simulink model "pasp_16i16c128s_part"  (Simulink 7.0)
settings: Solver=ode45  StartTime=0.0  StopTime=10  RelTol=1e-3
undefined symbols (not defined in the file or in its folder): pfb_coeff_gen_calc
libraries: xbsIndex_r4 x2019, casper_library x163, xps_library x21, gavrt_library x2
(part 1 of 21)
settings Model: Creator=tfiliba  LastModifiedBy=tfiliba
settings DebuggingCC: SaveWithParameterizedLinksMsg=none  SignalResolutionControl=TryResolveAllWithWarning  MultiTaskDSMMsg=warning  MultiTaskCondExecSysMsg=none  StrictBusMsg=None
settings RTWCC: IncludeERTFirstTime=on
settings GRTTargetCC: TargetFcnLib=ansi_tfl_tmw.mat

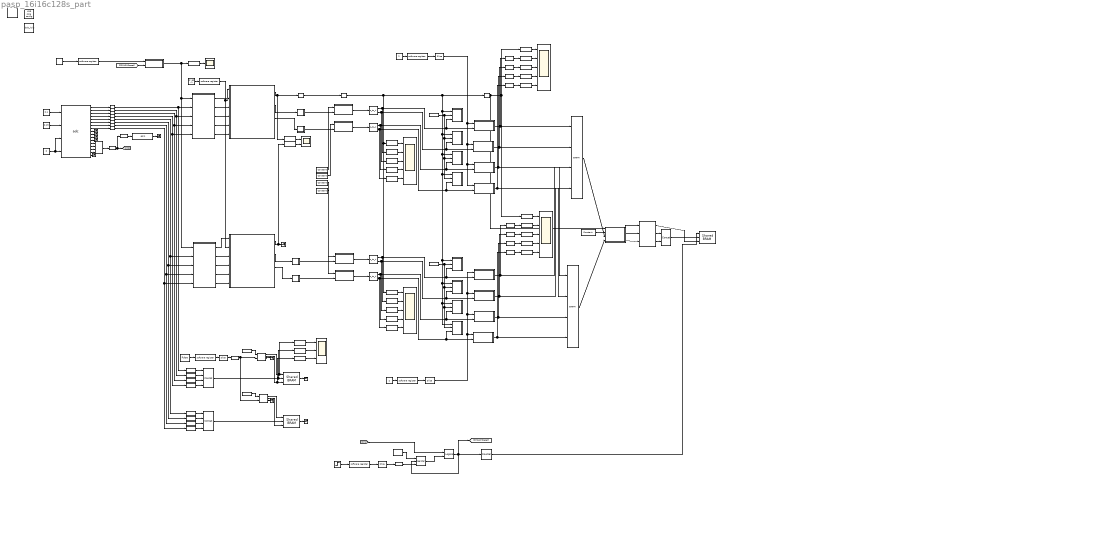
== system pasp_16i16c128s_part ==
  note: left
  note: Coherent-dedispersion Pulsar Machine
      | Nancay Radio Telescope
      | Peter McMahon (CASPER/meerKAT)
      | August 2008
      | Version 1.0
  note: ADC Scope
      | Outputs raw bits from ADC so you can measure voltage levels
  note: n * LCM(reoder_order=2)*(taps*clksperFFT=2*64=128)=256
  note: Pol 1
  note: Pol 2
  note: Real sampling at 800MSa/sec
      | to give B/W of 0-400MHz
  note: ADC Scope
      | Outputs raw bits from ADC so you can measure voltage levels
  note: ARM/PPS Reset Circuit
      | 1. User sets ARM high at half-second
      | 2. 1PPS arrives.
      | 3. PPSARMReset signal goes high for one clock on the 1PPS
      | 4. ARM is reset, and won't go high again until user brings it low then high
  note: Unsigned, 18-bits, b.p. at 12
      | Coefficient by which to scale the output of the FFT.
  note: Selects which 8 bits from 18 are outputted.
      | 0 is lowest 8-bits: bits 0-7 (inclusive)
      | 1: bits 4-9
      | 2: bits 8-15
      | 3 is highest 8-bits: bits 10-17
  note: Extract 8 bits from 18
  note: Extract 8 bits from 18
  note: Delay is here for timing purposes:
      | otherwise adc_sync fails timing.
  " System Generator" : xbsIndex_r4/ System Generator  Tag=genX  SystemSampleTime=-1  xilinxfamily=virtex2p  part=xc2vp50  speed=-7  package=ff1152  synthesis_tool=XST  clock_wrapper=Clock Enables  directory=./pasp_16i16c128s_part/sysgen  testbench=off  simulink_period=1  sysclk_period=5  dcm_input_clock_period=100  incr_netlist=off  trim_vbits=Everywhere in SubSystem  dbl_ovrd=According to Block Masks  core_generation=According to Block Masks  run_coregen=off  deprecated_control=off  eval_field=0  block_type=sysgen
      settings: block_version=10.1.3
  Concat1 : xbsIndex_r4/Concat  num_inputs=2  dbl_ovrd=off  block_type=concat
      settings: block_version=10.1.3
  Constant{,1,3..4} (4 alike) : xbsIndex_r4/Constant  arith_type=Unsigned  const=1  n_bits=18  bin_pt=12  explicit_period=on  period=1  equ=P=C  opselect=C  inp2=PCIN>>17  opr=+  inp1=P  carry=CIN  dbl_ovrd=off  block_type=constant
  Constant2 : Constant  Value=2  OutDataType=sfix(16)  OutScaling=2^0
  Constant5 : Constant  Value=2^32 - 1  OutDataType=sfix(16)  OutScaling=2^0
  Constant6 : Constant  Value=2  OutDataType=sfix(16)  OutScaling=2^0
  Gateway Out{1..5,8,10..19,21..30} (26 alike) : xbsIndex_r4/Gateway Out  hdl_port=off  timing_constraint=None  locs_specified=off  LOCs={}  block_type=gatewayout
      settings: infoedit="Gateway out block.  Converts Xilinx fixed point inputs into ouputs of type Simulink integer, double, or fixed point.<P><P>Hardware notes:  In hardware these blocks become top level output ports or are discarded, depending on how they are configured."  xl_area=[0, 0, 0, 0, 0, 0, 0]  block_version=10.1.3
  Reinterpret{,1..3,8..11} (8 alike) : xbsIndex_r4/Reinterpret  force_arith_type=on  arith_type="Signed  (2's comp)"  force_bin_pt=on  bin_pt=0  block_type=reinterpret
      settings: infoedit="Changes signal type without altering the binary representation.   You can changed the signal between signed and unsigned, and relocate the binary point.<P><P>Hardware notes: In hardware this block costs nothing.<P><P>Example:  Suppose the input is 6 bits wide, signed, with 2 fractional bits, and the output is forced to unsigned with 0 fractional bits.  Then an input of -2.0 (1110.00 in binary 2's complement) becomes an output of 56 (111000 in binary)."  block_version=10.1.3
  Scope : Scope  NumInputPorts=3
      settings: YMin=-5~-5~-5  YMax=5~5~5  SaveName=ScopeData8
  Scope1 : Scope  NumInputPorts=2
      settings: YMin=-1~-1  YMax=1~1  SaveName=ScopeData7
  Scope2 : Scope
      settings: YMin=-1  YMax=1  SaveName=ScopeData9
  Scope{3..6} (4 alike) : Scope  NumInputPorts=5
      Scope3 settings: YMin=-1~-2.5e-005~-1.75e-005~-2.5e-005~-8e-006  YMax=1~0~0~0~0  SaveName=ScopeData3
      Scope4 settings: YMin=-1~-1~-1~-1~-1  YMax=1~0~0~0~0  SaveName=ScopeData10
      Scope5 settings: YMin=-1~-2.5e-005~-1.75e-005~-2.5e-005~-8e-006  YMax=1~0~0~0~0  SaveName=ScopeData5
      Scope6 settings: YMin=-1~-1~-1~-1~-1  YMax=1~0~0~0~0  SaveName=ScopeData11
  Slice10 : xbsIndex_r4/Slice  nbits=2  boolean_output=off  mode=Lower Bit Location + Width  bit1=0  base1=MSB of Input  bit0=0  base0=LSB of Input  dbl_ovrd=off  block_type=slice
      settings: infoedit="Extracts a given range of bits from each input sample and presents it at the output.  The output type is ordinarily unsigned with binary point at zero, but can be Boolean when the slice is one bit wide.<P><P>Hardware notes: In hardware this block costs nothing."  block_version=10.1.3
  Slice2 : xbsIndex_r4/Slice  nbits=2  boolean_output=off  mode=Lower Bit Location + Width  bit1=0  base1=MSB of Input  bit0=0  base0=LSB of Input  dbl_ovrd=off  block_type=slice
      settings: infoedit="Extracts a given range of bits from each input sample and presents it at the output.  The output type is ordinarily unsigned with binary point at zero, but can be Boolean when the slice is one bit wide.<P><P>Hardware notes: In hardware this block costs nothing."  block_version=10.1.3
  Slice8 : xbsIndex_r4/Slice  nbits=1  boolean_output=on  mode=Lower Bit Location + Width  bit1=0  base1=MSB of Input  bit0=0  base0=LSB of Input  dbl_ovrd=off  block_type=slice
      settings: infoedit="Extracts a given range of bits from each input sample and presents it at the output.  The output type is ordinarily unsigned with binary point at zero, but can be Boolean when the slice is one bit wide.<P><P>Hardware notes: In hardware this block costs nothing."  block_version=10.1.3
  Terminator{,1..9,11} (11 alike) : Terminator
  XSG core config : xps_library/XSG core config  Tag=xps:xsg  SystemSampleTime=-1  hw_sys=iBOB  ibob_linux=off  clk_src=adc0_clk  iBOB_clk_src=adc0_clk  BEE2_ctrl_clk_src=sys_clk  BEE2_usr_clk_src=sys_clk  ROACH_clk_src=sys_clk  CORR_clk_src=sys_clk  gpio_clk_io_group=iBOB:sma  gpio_clk_bit_index=0  clk_rate=200  sample_period=1  synthesis_tool=XST  mpc_type=powerpc405
  adc : xps_library/adc  Tag=xps:adc  SystemSampleTime=-1  adc_brd=adc0  adc_clk_rate=800  adc_interleave=off  sample_period=1
  adc_data_delay{1..8} (8 alike) : xbsIndex_r4/Delay  en=off  latency=1  dbl_ovrd=off  reg_retiming=on  block_type=delay
      settings: xl_area=[0, 0, 0, 0, 0, 0, 0]
  bitselect_1 : Subsystem
  bitselect_2 : Subsystem
  bitselect_3 : Subsystem
  bitselect_4 : Subsystem
  bitselect_5 : Subsystem
  bitselect_6 : Subsystem
  bitselect_7 : Subsystem
  bitselect_8 : Subsystem
  bram_adcscope_1 : xps_library/Shared BRAM  Tag=xps:bram  SystemSampleTime=-1  arith_type="Signed  (2's comp)"  addr_width=11  data_width=32  data_bin_pt=0  init_vals=[1:2^11]  sample_rate=1
      link override convert_din: arith_type="Signed  (2's comp)"
      link override mem/sim_data_in: arith_type="Signed  (2's comp)"
      link override pasp_16i16c128s_part_bram_adcscope_1_data_out: arith_type="Signed  (2's comp)"
      link override pasp_16i16c128s_pasp_bram_adcscope_1_data_out: arith_type="Signed  (2's comp)"
  bram_adcscope_2 : xps_library/Shared BRAM  Tag=xps:bram  SystemSampleTime=-1  arith_type="Signed  (2's comp)"  addr_width=11  data_width=32  data_bin_pt=0  init_vals=[1:2^11]  sample_rate=1
      link override convert_din: arith_type="Signed  (2's comp)"
      link override mem/sim_data_in: arith_type="Signed  (2's comp)"
      link override pasp_16i16c128s_part_bram_adcscope_2_data_out: arith_type="Signed  (2's comp)"
      link override pasp_16i16c128s_pasp_bram_adcscope_1_data_out: arith_type="Signed  (2's comp)"
  bram_adcscope_3 : xps_library/Shared BRAM  Tag=xps:bram  SystemSampleTime=-1  arith_type="Signed  (2's comp)"  addr_width=11  data_width=32  data_bin_pt=0  init_vals=[1:2^11]  sample_rate=1
      link override convert_din: arith_type="Signed  (2's comp)"
      link override mem/sim_data_in: arith_type="Signed  (2's comp)"
      link override pasp_16i16c128s_part_bram_adcscope_3_data_out: arith_type="Signed  (2's comp)"
      link override pasp_16i16c128s_pasp_bram_adcscope_2_data_out: arith_type="Signed  (2's comp)"
  bs_delay : xbsIndex_r4/Delay  en=off  latency=1  dbl_ovrd=off  reg_retiming=off  block_type=delay
      settings: xl_area=[0, 0, 0, 0, 0, 0, 0]
  c_to_ri{1..4} (4 alike) : casper_library/Misc/c_to_ri  SystemSampleTime=-1  n_bits=18  bin_pt=17
      settings: AttributesFormatString=18_17 r/i
  cns_adcscope_1 : xbsIndex_r4/Constant  arith_type=Boolean  const=1  n_bits=1  bin_pt=0  explicit_period=on  period=1  equ=P=C  opselect=C  inp2=PCIN>>17  opr=+  inp1=P  carry=CIN  dbl_ovrd=off  block_type=constant
  cns_adcscope_2 : xbsIndex_r4/Constant  arith_type=Boolean  const=1  n_bits=1  bin_pt=0  explicit_period=on  period=1  equ=P=C  opselect=C  inp2=PCIN>>17  opr=+  inp1=P  carry=CIN  dbl_ovrd=off  block_type=constant
  cns_reorder : xbsIndex_r4/Constant  arith_type=Boolean  const=1  n_bits=16  bin_pt=14  explicit_period=on  period=1  equ=P=C  opselect=C  inp2=PCIN>>17  opr=+  inp1=P  carry=CIN  dbl_ovrd=off  block_type=constant
  cns_reset : xbsIndex_r4/Constant  arith_type=Boolean  const=1  n_bits=1  bin_pt=0  explicit_period=on  period=1  equ=P=C  opselect=C  inp2=PCIN>>17  opr=+  inp1=P  carry=CIN  dbl_ovrd=off  block_type=constant
  cns_scopes_en0 : xbsIndex_r4/Constant  arith_type=Boolean  const=1  n_bits=1  bin_pt=0  explicit_period=on  period=1  equ=P=C  opselect=C  inp2=PCIN>>17  opr=+  inp1=P  carry=CIN  dbl_ovrd=off  block_type=constant
  cns_scopes_en1 : xbsIndex_r4/Constant  arith_type=Boolean  const=1  n_bits=1  bin_pt=0  explicit_period=on  period=1  equ=P=C  opselect=C  inp2=PCIN>>17  opr=+  inp1=P  carry=CIN  dbl_ovrd=off  block_type=constant
  cns_sync_period : Constant  Value=10240  OutDataType=sfix(16)  OutScaling=2^0
  cnt_adcscope_1 : casper_library/Misc/freeze_cntr  SystemSampleTime=-1  CounterBits=11
  cnt_adcscope_2 : casper_library/Misc/freeze_cntr  SystemSampleTime=-1  CounterBits=11
  coeff_delay : xbsIndex_r4/Delay  en=off  latency=6  dbl_ovrd=off  reg_retiming=on  block_type=delay
      settings: xl_area=[0, 0, 0, 0, 0, 0, 0]
  concat_adcscope_1 : xbsIndex_r4/Concat  num_inputs=4  dbl_ovrd=off  block_type=concat
      settings: block_version=10.1.3
  concat_adcscope_3 : xbsIndex_r4/Concat  num_inputs=4  dbl_ovrd=off  block_type=concat
      settings: block_version=10.1.3
  cram0 : gavrt_library/cram  SystemSampleTime=-1  num_slice=4
  cram1 : gavrt_library/cram  SystemSampleTime=-1  num_slice=4
  fft_delay : xbsIndex_r4/Delay  en=off  latency=4  dbl_ovrd=off  reg_retiming=off  block_type=delay
      settings: xl_area=[0, 0, 0, 0, 0, 0, 0]
  fft_wideband_real : Subsystem  AncestorBlock=casper_library/FFTs/fft_wideband_real  mask(fft_wideband_real){FFTSize=5, input_bit_width=18, coeff_bit_width=18, n_inputs=2, quantization=Truncate, overflow=Wrap, add_latency=2, mult_latency=3, bram_latency=2, arch=Virtex2Pro, opt_target=Multipliers, coeffs_bit_limit=2, delays_bit_limit=8, specify_mult=on, mult_spec=[1 1 1 1 1]}
      mask prompts: FFTSize "Size of FFT: (2^?)"; input_bit_width "Input/Output Bit Width"; coeff_bit_width "Coefficient Bit Width"; n_inputs "Number of Simultaneous Inputs: (2^?)"; quantization "Quantization Behavior"; overflow "Overflow Behavior"; add_latency "Add Latency"; mult_latency "Mult Latency"; bram_latency "BRAM Latency"; arch "Architecture"; opt_target "Optimisation Target"; coeffs_bit_limit "Number bits above which to store stage's coefficients in BRAM (2^? bits)"; delays_bit_limit "Number bits above which to store stage's delays in BRAM (2^? bits)"; specify_mult "Specify multiplier use (behavioral HDL if not specified)"; mult_spec "Multiplier specification (0=core, 1=embedded, 2=behavioural) (left=1st stage)"
      mask init:
      | fft_wideband_real_init(gcb,...
      |     'FFTSize', FFTSize,...
      |     'input_bit_width', input_bit_width,...
      |     'coeff_bit_width', coeff_bit_width,...
      |     'n_inputs', n_inputs,...
      |     'quantization', quantization,...
      |     'overflow', overflow,...
      |     'add_latency', add_latency,...
      |     'mult_latency', mult_latency,...
      |     'bram_latency', bram_latency,...
      |     'arch', arch, ...
      |     'opt_target', opt_target, ...
      |     'coeffs_bit_limit', coeffs_bit_limit, ...
      |     'delays_bit_limit', delays_bit_limit, ...    
      |     'specify_mult', specify_mult,...
      |     'mult_spec', mult_spec);
      settings: AttributesFormatString=FFTSize=5
      mask Help: eval('xlWeb([getenv(''MLIB_ROOT''), ''\doc\htmlblocks\sp_fft_wideband_real\sp_fft_wideband_real.html''])')
      mask StyleString: edit,edit,edit,edit,popup(Truncate|Round  (unbiased: +/- Inf)|Round  (unbiased: Even Values)),popup(Wrap|Saturate|Error),edit,edit,edit,popup(Virtex2Pro|Virtex5),popup(Logic|Multipliers),edit,edit,checkbox,edit
      mask CallbackString:
      | % fft_size = eval(get_param(gcb, 'FFTSize'));
      | % old_vec = eval(get_param(gcb, 'mult_spec'));
      | 
      | % vec = 2.*ones(1,fft_size);
      | % old_size = length(old_vec);
      | 
      | % if(old_size <= fft_size),
      | %    size = old_size;
      | % else,
      | %     size = fft_size;
      | % end
      | 
      | % for n = 1:size, vec(n) = old_vec(n); end
      | % set_param(gcb, 'mult_spec', mat2str(vec));|||||||||||||specify_mult = get_param(gcb,'specify_mult');
      | visibs = get_param(gcb, 'MaskVisibilities');
      | enables = get_param(gcb, 'MaskEnables');
      | enables{15} = 'on';
      | if( strcmp(specify_mult,'off')), visibs{15} = 'off';
      | else, visibs{15} = 'on';
      | end
      | set_param(gcb, 'MaskEnables', enables);
      | set_param(gcb, 'MaskVisibilities', visibs);
      | |% vec = eval( get_param(gcb, 'mult_spec'));
      | % vec_size = length(vec);
      | % fft_size = eval( get_param(gcb, 'FFTSize'));
      | % new_vec = 2.*ones(1, fft_size);
      | 
      | % if(vec_size <= fft_size), size = vec_size;
      | % else size = fft_size;
      | % end
      | 
      | % for n=1:size
      | %     new_vec(n) = vec(n);
      | % end
      | % set_param(gcb, 'mult_spec', mat2str(new_vec));
  fft_wideband_real1 : Subsystem  AncestorBlock=casper_library/FFTs/fft_wideband_real  mask(fft_wideband_real){FFTSize=5, input_bit_width=18, coeff_bit_width=18, n_inputs=2, quantization=Truncate, overflow=Wrap, add_latency=2, mult_latency=3, bram_latency=2, arch=Virtex2Pro, opt_target=Multipliers, coeffs_bit_limit=2, delays_bit_limit=8, specify_mult=on, mult_spec=[1 1 1 1 1]}
      mask prompts: FFTSize "Size of FFT: (2^?)"; input_bit_width "Input/Output Bit Width"; coeff_bit_width "Coefficient Bit Width"; n_inputs "Number of Simultaneous Inputs: (2^?)"; quantization "Quantization Behavior"; overflow "Overflow Behavior"; add_latency "Add Latency"; mult_latency "Mult Latency"; bram_latency "BRAM Latency"; arch "Architecture"; opt_target "Optimisation Target"; coeffs_bit_limit "Number bits above which to store stage's coefficients in BRAM (2^? bits)"; delays_bit_limit "Number bits above which to store stage's delays in BRAM (2^? bits)"; specify_mult "Specify multiplier use (behavioral HDL if not specified)"; mult_spec "Multiplier specification (0=core, 1=embedded, 2=behavioural) (left=1st stage)"
      mask init: same as pasp_16i16c128s_part/fft_wideband_real
      settings: AttributesFormatString=FFTSize=5
      mask Help: eval('xlWeb([getenv(''MLIB_ROOT''), ''\doc\htmlblocks\sp_fft_wideband_real\sp_fft_wideband_real.html''])')
      mask StyleString: edit,edit,edit,edit,popup(Truncate|Round  (unbiased: +/- Inf)|Round  (unbiased: Even Values)),popup(Wrap|Saturate|Error),edit,edit,edit,popup(Virtex2Pro|Virtex5),popup(Logic|Multipliers),edit,edit,checkbox,edit
      mask CallbackString:
      | % fft_size = eval(get_param(gcb, 'FFTSize'));
      | % old_vec = eval(get_param(gcb, 'mult_spec'));
      | 
      | % vec = 2.*ones(1,fft_size);
      | % old_size = length(old_vec);
      | 
      | % if(old_size <= fft_size),
      | %    size = old_size;
      | % else,
      | %     size = fft_size;
      | % end
      | 
      | % for n = 1:size, vec(n) = old_vec(n); end
      | % set_param(gcb, 'mult_spec', mat2str(vec));|||||||||||||specify_mult = get_param(gcb,'specify_mult');
      | visibs = get_param(gcb, 'MaskVisibilities');
      | enables = get_param(gcb, 'MaskEnables');
      | enables{15} = 'on';
      | if( strcmp(specify_mult,'off')), visibs{15} = 'off';
      | else, visibs{15} = 'on';
      | end
      | set_param(gcb, 'MaskEnables', enables);
      | set_param(gcb, 'MaskVisibilities', visibs);
      | |% vec = eval( get_param(gcb, 'mult_spec'));
      | % vec_size = length(vec);
      | % fft_size = eval( get_param(gcb, 'FFTSize'));
      | % new_vec = 2.*ones(1, fft_size);
      | 
      | % if(vec_size <= fft_size), size = vec_size;
      | % else size = fft_size;
      | % end
      | 
      | % for n=1:size
      | %     new_vec(n) = vec(n);
      | % end
      | % set_param(gcb, 'mult_spec', mat2str(new_vec));
  force_adc_i{0..3,8..11} (8 alike) : xbsIndex_r4/Reinterpret  force_arith_type=on  arith_type=Unsigned  force_bin_pt=on  bin_pt=0  block_type=reinterpret
      settings: infoedit="Changes signal type without altering the binary representation.   You can changed the signal between signed and unsigned, and relocate the binary point.<P><P>Hardware notes: In hardware this block costs nothing.<P><P>Example:  Suppose the input is 6 bits wide, signed, with 2 fractional bits, and the output is forced to unsigned with 0 fractional bits.  Then an input of -2.0 (1110.00 in binary 2's complement) becomes an output of 56 (111000 in binary)."  block_version=10.1.3
  ibob_lwip : xps_library/ibob_lwip  SystemSampleTime=-1
      link override macbits/convert: arith_type=Unsigned  n_bits=32  bin_pt=0
      link override machdr/pasp_16i16c128s_ibob_lwip_machdr_gateway: arith_type=Unsigned  n_bits=20  bin_pt=0  period=sample_period
      link override machdr/pasp_16i16c128s_part_ibob_lwip_machdr_gateway: arith_type=Unsigned  n_bits=20  bin_pt=0  period=sample_period
  led2_pps : xps_library/gpio  Tag=xps:gpio  SystemSampleTime=-1  io_group=iBOB:led  io_dir=out  arith_type=Boolean  bitwidth=1  bin_pt=0  bit_index=3  sample_period=1  use_single_ended=off  use_ddr=off  reg_iob=on  reg_clk_phase=0  termination=None
      link override convert: arith_type=Boolean  n_bits=1  bin_pt=0
  log_arm_pps : xbsIndex_r4/Logical  logical_function=AND  inputs=2  en=off  latency=0  precision=Full  arith_type=Unsigned  n_bits=8  bin_pt=2  align_bp=on  dbl_ovrd=off  block_type=logical
      settings: xl_area=[0, 0, 0, 0, 0, 0, 0]
  pfb_fir_real : Subsystem  AncestorBlock=casper_library/PFBs/pfb_fir_real  mask(pfb_fir_real){PFBSize=5, TotalTaps=2, WindowType=hamming, n_inputs=2, MakeBiplex=0, BitWidthIn=8, BitWidthOut=18, CoeffBitWidth=8, CoeffDistMem=0, add_latency=1, mult_latency=2, bram_latency=2, quantization=Truncate, fwidth=1, specify_mult=off, mult_spec=[0 1]}
      mask prompts: PFBSize "Size of PFB: (2^? pnts)"; TotalTaps "Total Number of Taps:"; WindowType "Windowing Function:"; n_inputs "Number of Simultaneous Inputs: (2^?)"; MakeBiplex "Make Biplex"; BitWidthIn "Input Bitwidth:"; BitWidthOut "Output Bitwidth:"; CoeffBitWidth "Coefficient Bitwidth:"; CoeffDistMem "Use Distributed Memory for Coeffs"; add_latency "Add Latency"; mult_latency "Mult Latency"; bram_latency "BRAM Latency"; quantization "Quantization Behavior"; fwidth "Bin Width Scaling (normal=1)"; specify_mult "Specify multiplier use (embedded if not specified)"; mult_spec "Multiplier specification (0=core, 1=embedded, 2=behavioural) (left=1st tap)"
      mask init:
      | pfb_fir_real_init(gcb,...
      |     'PFBSize', PFBSize,...
      |     'TotalTaps', TotalTaps,...
      |     'WindowType', WindowType,...
      |     'n_inputs', n_inputs,...
      |     'MakeBiplex', MakeBiplex,...
      |     'BitWidthIn', BitWidthIn,...
      |     'BitWidthOut', BitWidthOut,...
      |     'CoeffBitWidth', CoeffBitWidth,...
      |     'CoeffDistMem', CoeffDistMem,...
      |     'add_latency', add_latency,...
      |     'mult_latency', mult_latency,...
      |     'bram_latency', bram_latency,...
      |     'quantization', quantization,...
      |     'fwidth', fwidth,...
      |     'specify_mult', specify_mult,...
      |     'mult_spec', mult_spec);
      settings: AttributesFormatString=taps=2, add_latency=1
      mask Help: eval('xlWeb([getenv(''MLIB_ROOT''), ''\doc\htmlblocks\sp_pfb_fir_real\sp_pfb_fir_real.html''])')
      mask StyleString: edit,edit,popup(bartlett|barthannwin|blackman|blackmanharris|bohamwin|chebwin|flattopwin|gausswin|hamming|hann|kaiser|nuttallwin|parzenwin|rectwin|tukeywin|triang|userwindow),edit,edit,edit,edit,edit,edit,edit,edit,edit,popup(Truncate|Round  (unbiased: +/- Inf)|Round  (unbiased: Even Values)),edit,checkbox,edit
      mask CallbackString:
      | |num_taps = eval(get_param(gcb, 'TotalTaps'));
      | old_vec = eval(get_param(gcb, 'mult_spec'));
      | 
      | vec = ones(1,num_taps);
      | old_size = length(old_vec);
      | 
      | if(old_size <= num_taps),
      |     size = old_size;
      | else,
      |     size = num_taps;
      | end
      | 
      | for n = 1:size, vec(n) = old_vec(n); end
      | set_param(gcb, 'mult_spec', mat2str(vec));|||||||||||||specify_mult = get_param(gcb,'specify_mult');
      | if( strcmp(specify_mult,'off')),
      |     set_param(gcb, 'MaskEnables', {'on','on','on','on','on','on','on','on','on','on','on','on','on','on','on','off'});
      | else
      |     set_param(gcb, 'MaskEnables', {'on','on','on','on','on','on','on','on','on','on','on','on','on','on','on','on'});
      | end
      | 
      | |vec = eval( get_param(gcb, 'mult_spec'));
      | vec_size = length(vec);
      | num_taps = eval( get_param(gcb, 'TotalTaps'));
      | new_vec = ones(1, num_taps);
      | 
      | if(vec_size <= num_taps), size = vec_size;
      | else size = num_taps;
      | end
      | 
      | for n=1:size
      |     new_vec(n) = vec(n);
      | end
      | set_param(gcb, 'mult_spec', mat2str(new_vec));
  pfb_fir_real1 : Subsystem  AncestorBlock=casper_library/PFBs/pfb_fir_real  mask(pfb_fir_real){PFBSize=5, TotalTaps=2, WindowType=hamming, n_inputs=2, MakeBiplex=0, BitWidthIn=8, BitWidthOut=18, CoeffBitWidth=8, CoeffDistMem=0, add_latency=1, mult_latency=2, bram_latency=2, quantization=Truncate, fwidth=1, specify_mult=off, mult_spec=[0 1]}
      mask prompts: PFBSize "Size of PFB: (2^? pnts)"; TotalTaps "Total Number of Taps:"; WindowType "Windowing Function:"; n_inputs "Number of Simultaneous Inputs: (2^?)"; MakeBiplex "Make Biplex"; BitWidthIn "Input Bitwidth:"; BitWidthOut "Output Bitwidth:"; CoeffBitWidth "Coefficient Bitwidth:"; CoeffDistMem "Use Distributed Memory for Coeffs"; add_latency "Add Latency"; mult_latency "Mult Latency"; bram_latency "BRAM Latency"; quantization "Quantization Behavior"; fwidth "Bin Width Scaling (normal=1)"; specify_mult "Specify multiplier use (embedded if not specified)"; mult_spec "Multiplier specification (0=core, 1=embedded, 2=behavioural) (left=1st tap)"
      mask init: same as pasp_16i16c128s_part/pfb_fir_real
      settings: AttributesFormatString=taps=2, add_latency=1
      mask Help: eval('xlWeb([getenv(''MLIB_ROOT''), ''\doc\htmlblocks\sp_pfb_fir_real\sp_pfb_fir_real.html''])')
      mask StyleString: edit,edit,popup(bartlett|barthannwin|blackman|blackmanharris|bohamwin|chebwin|flattopwin|gausswin|hamming|hann|kaiser|nuttallwin|parzenwin|rectwin|tukeywin|triang|userwindow),edit,edit,edit,edit,edit,edit,edit,edit,edit,popup(Truncate|Round  (unbiased: +/- Inf)|Round  (unbiased: Even Values)),edit,checkbox,edit
      mask CallbackString:
      | |num_taps = eval(get_param(gcb, 'TotalTaps'));
      | old_vec = eval(get_param(gcb, 'mult_spec'));
      | 
      | vec = ones(1,num_taps);
      | old_size = length(old_vec);
      | 
      | if(old_size <= num_taps),
      |     size = old_size;
      | else,
      |     size = num_taps;
      | end
      | 
      | for n = 1:size, vec(n) = old_vec(n); end
      | set_param(gcb, 'mult_spec', mat2str(vec));|||||||||||||specify_mult = get_param(gcb,'specify_mult');
      | if( strcmp(specify_mult,'off')),
      |     set_param(gcb, 'MaskEnables', {'on','on','on','on','on','on','on','on','on','on','on','on','on','on','on','off'});
      | else
      |     set_param(gcb, 'MaskEnables', {'on','on','on','on','on','on','on','on','on','on','on','on','on','on','on','on'});
      | end
      | 
      | |vec = eval( get_param(gcb, 'mult_spec'));
      | vec_size = length(vec);
      | num_taps = eval( get_param(gcb, 'TotalTaps'));
      | new_vec = ones(1, num_taps);
      | 
      | if(vec_size <= num_taps), size = vec_size;
      | else size = num_taps;
      | end
      | 
      | for n=1:size
      |     new_vec(n) = vec(n);
      | end
      | set_param(gcb, 'mult_spec', mat2str(new_vec));
  pipeline : Subsystem  AncestorBlock=casper_library/Delays/pipeline  mask(pipeline){latency=4}
      mask help:
      | An explicitly laid-out delay line to avoid mapping to SRL16s
      | for use in pipelining to help achieve timing closure.
      mask init:
      | pipeline_init(gcb, 'latency', latency);
      mask Display:
      | port_label('input', 1, 'd');
      | port_label('output', 1, 'q');
      | disp(['Z^-', num2str(latency)]);
  pipeline1 : Subsystem  AncestorBlock=casper_library/Delays/pipeline  mask(pipeline){latency=4}
      mask help:
      | An explicitly laid-out delay line to avoid mapping to SRL16s
      | for use in pipelining to help achieve timing closure.
      mask init:
      | pipeline_init(gcb, 'latency', latency);
      mask Display:
      | port_label('input', 1, 'd');
      | port_label('output', 1, 'q');
      | disp(['Z^-', num2str(latency)]);
  pipeline2 : Subsystem  AncestorBlock=casper_library/Delays/pipeline  mask(pipeline){latency=4}
      mask help:
      | An explicitly laid-out delay line to avoid mapping to SRL16s
      | for use in pipelining to help achieve timing closure.
      mask init:
      | pipeline_init(gcb, 'latency', latency);
      mask Display:
      | port_label('input', 1, 'd');
      | port_label('output', 1, 'q');
      | disp(['Z^-', num2str(latency)]);
  pipeline3 : Subsystem  AncestorBlock=casper_library/Delays/pipeline  mask(pipeline){latency=4}
      mask help:
      | An explicitly laid-out delay line to avoid mapping to SRL16s
      | for use in pipelining to help achieve timing closure.
      mask init:
      | pipeline_init(gcb, 'latency', latency);
      mask Display:
      | port_label('input', 1, 'd');
      | port_label('output', 1, 'q');
      | disp(['Z^-', num2str(latency)]);
  posedge_adcscope : casper_library/Misc/posedge  Tag=Rising Edge Detector  SystemSampleTime=-1
  posedge_arm : casper_library/Misc/posedge  Tag=Rising Edge Detector  SystemSampleTime=-1
  posedge_pps : casper_library/Misc/posedge  Tag=Rising Edge Detector  SystemSampleTime=-1
  pulse_ext : casper_library/Misc/pulse_ext  SystemSampleTime=-1  pulse_len=10000000
  reg_adcscope_trigger : xps_library/software register  Tag=xps:sw_reg  SystemSampleTime=-1  io_dir=From Processor  arith_type=Unsigned  bitwidth=32  bin_pt=0  sample_period=1  gw_name=pasp_16i16c128s_part_reg_adcscope_trigger_user_data_out
      link override pasp_16i16c128s_part_reg_adcscope_trigger_user_data_out: arith_type=Unsigned  n_bits=32  bin_pt=0  period=1
      link override pasp_16i16c128s_pasp_reg_adcscope_trigger_user_data_out: arith_type=Unsigned  n_bits=32  bin_pt=0  period=1
  reg_arm : xps_library/software register  Tag=xps:sw_reg  SystemSampleTime=-1  io_dir=From Processor  arith_type=Unsigned  bitwidth=32  bin_pt=0  sample_period=1  gw_name=pasp_16i16c128s_part_reg_arm_user_data_out
      link override pasp_16i16c128s_part_reg_arm_user_data_out: arith_type=Unsigned  n_bits=32  bin_pt=0  period=1
      link override pasp_16i16c128s_pasp_reg_arm_user_data_out: arith_type=Unsigned  n_bits=32  bin_pt=0  period=1
  reg_fft_shift : xps_library/software register  Tag=xps:sw_reg  SystemSampleTime=-1  io_dir=From Processor  arith_type=Unsigned  bitwidth=32  bin_pt=0  sample_period=1  gw_name=pasp_16i16c128s_part_reg_fft_shift_user_data_out
      link override pasp_16i16c128s_part_reg_fft_shift_user_data_out: arith_type=Unsigned  n_bits=32  bin_pt=0  period=1
      link override pasp_16i16c128s_pasp_reg_fft_shift_user_data_out: arith_type=Unsigned  n_bits=32  bin_pt=0  period=1
  reg_output_bitselect_pol1 : xps_library/software register  Tag=xps:sw_reg  SystemSampleTime=-1  io_dir=From Processor  arith_type=Unsigned  bitwidth=32  bin_pt=0  sample_period=1  gw_name=pasp_16i16c128s_part_reg_output_bitselect_pol1_user_data_out
      link override pasp_16i16c128s_part_reg_output_bitselect_pol1_user_data_out: arith_type=Unsigned  n_bits=32  bin_pt=0  period=1
      link override pasp_16i16c128s_pasp_reg_output_bitselect_pol1_user_data_out: arith_type=Unsigned  n_bits=32  bin_pt=0  period=1
  reg_output_bitselect_pol2 : xps_library/software register  Tag=xps:sw_reg  SystemSampleTime=-1  io_dir=From Processor  arith_type=Unsigned  bitwidth=32  bin_pt=0  sample_period=1  gw_name=pasp_16i16c128s_part_reg_output_bitselect_pol2_user_data_out
      link override pasp_16i16c128s_part_reg_output_bitselect_pol2_user_data_out: arith_type=Unsigned  n_bits=32  bin_pt=0  period=1
      link override pasp_16i16c128s_pasp_reg_output_bitselect_pol2_user_data_out: arith_type=Unsigned  n_bits=32  bin_pt=0  period=1
  reg_reset_arm : xbsIndex_r4/Register  init=0  rst=on  en=on  dbl_ovrd=off  block_type=register
      settings: xl_area=[0, 0, 0, 0, 0, 0, 0]
  reg_sync_period : xps_library/software register  Tag=xps:sw_reg  SystemSampleTime=-1  io_dir=From Processor  arith_type=Unsigned  bitwidth=32  bin_pt=0  sample_period=1  gw_name=pasp_16i16c128s_part_reg_sync_period_user_data_out
      link override pasp_16i16c128s_part_reg_sync_period_user_data_out: arith_type=Unsigned  n_bits=32  bin_pt=0  period=1
      link override pasp_16i16c128s_pasp_reg_sync_period_user_data_out: arith_type=Unsigned  n_bits=32  bin_pt=0  period=1
  reorder : Subsystem  AncestorBlock=casper_library/Reorder/reorder  mask(reorder){map=[0 8 1 9 2 10 3 11 4 12 5 13 6 14 7 15], n_inputs=2, bram_latency=2, map_latency=3, double_buffer=1}
      mask prompts: map "Output Order:"; n_inputs "Number of inputs"; bram_latency "BRAM Latency"; map_latency "Map Latency"; double_buffer "Double Buffer"
      mask init:
      | reorder_init(gcb, ...
      |     'map', map, ...
      |     'n_inputs', n_inputs, ...
      |     'bram_latency', bram_latency, ...
      |     'map_latency', map_latency, ...
      |     'double_buffer', double_buffer);
      settings: AttributesFormatString=order=2
      mask Help: eval('xlWeb([getenv(''MLIB_ROOT''), ''\doc\htmlblocks\sp_reorder\sp_reorder.html''])')
  scale1 : Subsystem
  scale2 : Subsystem
  scale3 : Subsystem
  scale4 : Subsystem
  scope_output1 : Subsystem
  scope_output2 : Subsystem
  scope_output3 : Subsystem
  scope_output4 : Subsystem
  scope_output5 : Subsystem
  scope_output6 : Subsystem
  scope_output7 : Subsystem
  scope_output8 : Subsystem
  sim_adcscope_trigger : DiscretePulseGenerator  Period=5000
  sim_cns : Constant  Value=0.1  OutDataType=sfix(16)  OutScaling=2^0
  sim_cns1 : Constant  Value=0.15  OutDataType=sfix(16)  OutScaling=2^0
  sim_step_arm : Step  SampleTime=0
  sim_zero : Constant  Value=0  OutDataType=sfix(16)  OutScaling=2^0
  slc_arm : xbsIndex_r4/Slice  nbits=1  boolean_output=on  mode=Lower Bit Location + Width  bit1=0  base1=MSB of Input  bit0=0  base0=LSB of Input  dbl_ovrd=off  block_type=slice
      settings: infoedit="Extracts a given range of bits from each input sample and presents it at the output.  The output type is ordinarily unsigned with binary point at zero, but can be Boolean when the slice is one bit wide.<P><P>Hardware notes: In hardware this block costs nothing."  block_version=10.1.3
  square_transposer : casper_library/Reorder/square_transposer  SystemSampleTime=-1  n_inputs=1
      settings: AttributesFormatString=n_inputs=1
  sync_generator : Subsystem
  sync_or : xbsIndex_r4/Logical  logical_function=OR  inputs=4  en=off  latency=1  precision=Full  arith_type=Unsigned  n_bits=8  bin_pt=2  align_bp=on  dbl_ovrd=off  block_type=logical
      settings: xl_area=[0, 0, 0, 0, 0, 0, 0]
  system_counter : xbsIndex_r4/Counter  cnt_type=Free Running  cnt_to=Inf  operation=Up  start_count=0  cnt_by_val=1  arith_type=Unsigned  n_bits=64  bin_pt=0  load_pin=off  rst=on  en=off  explicit_period=off  period=1  dbl_ovrd=off  use_behavioral_HDL=off  use_rpm=on  implementation=Fabric  block_type=counter
      settings: xl_area=[0, 0, 0, 0, 0, 0, 0]
  wires:
  reg_fft_shift/1 -> fft_wideband_real1/2(shift), fft_wideband_real/2(shift)
  fft_wideband_real/1(sync_out) -> fft_delay/1, Gateway Out4/1
  fft_wideband_real1/1(sync_out) -> Terminator/1, Gateway Out5/1
  reg_sync_period/1 -> sync_generator/1(period)
  sim_zero/1 -> adc/3, adc/4
  adc/1 -> adc_data_delay1/1
  sync_generator/1(sync) -> Gateway Out8/1, pfb_fir_real/1(sync), pfb_fir_real1/1(sync)
  adc/2 -> adc_data_delay2/1
  adc/3 -> adc_data_delay3/1
  adc/4 -> adc_data_delay4/1
  adc/5 -> adc_data_delay5/1
  adc/6 -> adc_data_delay6/1
  adc/7 -> adc_data_delay7/1
  adc/8 -> adc_data_delay8/1
  cns_sync_period/1 -> reg_sync_period/1
  adc/13 -> sync_or/1
  adc/14 -> sync_or/2
  adc/15 -> sync_or/3
  adc/16 -> sync_or/4
  sync_or/1 -> posedge_pps/1
  Gateway Out8/1 -> Scope2/1
  posedge_pps/1 -> [PPS], pulse_ext/1
  [PPSARMReset] -> sync_generator/2(reset)
  pulse_ext/1 -> led2_pps/1
  reg_arm/1 -> slc_arm/1
  cns_reset/1 -> reg_reset_arm/1
  reg_reset_arm/1 -> log_arm_pps/2
  [PPS] -> log_arm_pps/1
  log_arm_pps/1 -> reg_reset_arm/2, system_counter/1, [PPSARMReset]
  slc_arm/1 -> posedge_arm/1
  posedge_arm/1 -> reg_reset_arm/3
  concat_adcscope_1/1 -> bram_adcscope_1/2, Gateway Out2/1
  force_adc_i0/1 -> concat_adcscope_1/1
  force_adc_i1/1 -> concat_adcscope_1/2
  force_adc_i2/1 -> concat_adcscope_1/3
  force_adc_i3/1 -> concat_adcscope_1/4
  force_adc_i9/1 -> concat_adcscope_3/2
  force_adc_i10/1 -> concat_adcscope_3/3
  force_adc_i11/1 -> concat_adcscope_3/4
  sim_step_arm/1 -> reg_arm/1
  fft_wideband_real/2(out0) -> pipeline/1(d)
  coeff_delay/1 -> scope_output1/1(rst), scope_output2/1(rst), scope_output3/1(rst), scope_output5/1(rst), scope_output6/1(rst), scope_output7/1(rst), scope_output8/1(rst), scope_output4/1(rst), bs_delay/1, Gateway Out11/1, Gateway Out22/1
  scale1/1(data_out) -> c_to_ri1/1
  scale2/1(data_out) -> c_to_ri2/1
  fft_wideband_real1/2(out0) -> pipeline2/1(d)
  Constant2/1 -> reg_output_bitselect_pol1/1
  reg_output_bitselect_pol1/1 -> Slice2/1
  c_to_ri1/1 -> bitselect_1/2(data_in), scope_output1/3(data_in), Gateway Out12/1
  c_to_ri2/2 -> Gateway Out14/1, scope_output4/3(data_in), bitselect_4/2(data_in)
  c_to_ri2/1 -> Gateway Out13/1, scope_output3/3(data_in), bitselect_3/2(data_in)
  c_to_ri1/2 -> scope_output2/3(data_in), bitselect_2/2(data_in), Gateway Out10/1
  bitselect_1/1(data_out) -> Reinterpret/1, cram0/1
  bitselect_2/1(data_out) -> Reinterpret1/1, cram0/2
  bitselect_3/1(data_out) -> Reinterpret2/1, cram1/1
  bitselect_4/1(data_out) -> Reinterpret3/1, cram1/2
  Constant5/1 -> reg_fft_shift/1
  cnt_adcscope_1/1 -> bram_adcscope_1/1, Gateway Out1/1
  cnt_adcscope_1/2 -> bram_adcscope_1/3, Gateway Out3/1
  cns_adcscope_1/1 -> cnt_adcscope_1/1
  sim_adcscope_trigger/1 -> reg_adcscope_trigger/1
  reg_adcscope_trigger/1 -> Slice8/1
  posedge_adcscope/1 -> cnt_adcscope_1/2, cnt_adcscope_2/2
  concat_adcscope_3/1 -> bram_adcscope_3/2
  cnt_adcscope_2/1 -> bram_adcscope_3/1
  cnt_adcscope_2/2 -> bram_adcscope_3/3
  cns_adcscope_2/1 -> cnt_adcscope_2/1
  Gateway Out1/1 -> Scope/1
  Gateway Out2/1 -> Scope/2
  Gateway Out3/1 -> Scope/3
  cns_scopes_en0/1 -> scope_output1/2(en), scope_output2/2(en), scope_output3/2(en), scope_output4/2(en)
  Slice2/1 -> bitselect_1/1(bitselect), bitselect_3/1(bitselect), bitselect_4/1(bitselect), bitselect_2/1(bitselect)
  sim_cns/1 -> adc/1
  Gateway Out11/1 -> Scope3/1
  Gateway Out12/1 -> Scope3/2
  Gateway Out10/1 -> Scope3/3
  Gateway Out13/1 -> Scope3/4
  Gateway Out14/1 -> Scope3/5
  bs_delay/1 -> Gateway Out16/1, Gateway Out27/1, reorder/1(sync)
  Gateway Out16/1 -> Scope4/1
  Gateway Out17/1 -> Scope4/2
  Gateway Out15/1 -> Scope4/3
  Gateway Out18/1 -> Scope4/4
  Gateway Out19/1 -> Scope4/5
  Slice8/1 -> posedge_adcscope/1
  Reinterpret/1 -> Gateway Out17/1
  Reinterpret1/1 -> Gateway Out15/1
  Reinterpret2/1 -> Gateway Out18/1
  Reinterpret3/1 -> Gateway Out19/1
  c_to_ri3/1 -> scope_output5/3(data_in), bitselect_5/2(data_in), Gateway Out23/1
  c_to_ri4/2 -> Gateway Out25/1, scope_output8/3(data_in), bitselect_8/2(data_in)
  c_to_ri4/1 -> Gateway Out24/1, scope_output7/3(data_in), bitselect_7/2(data_in)
  c_to_ri3/2 -> scope_output6/3(data_in), bitselect_6/2(data_in), Gateway Out21/1
  bitselect_5/1(data_out) -> Reinterpret8/1, cram0/3
  bitselect_6/1(data_out) -> Reinterpret9/1, cram0/4
  bitselect_7/1(data_out) -> Reinterpret10/1, cram1/3
  bitselect_8/1(data_out) -> Reinterpret11/1, cram1/4
  cns_scopes_en1/1 -> scope_output5/2(en), scope_output6/2(en), scope_output7/2(en), scope_output8/2(en)
  Gateway Out22/1 -> Scope5/1
  Gateway Out23/1 -> Scope5/2
  Gateway Out21/1 -> Scope5/3
  Gateway Out24/1 -> Scope5/4
  Gateway Out25/1 -> Scope5/5
  scale3/1(data_out) -> c_to_ri3/1
  scale4/1(data_out) -> c_to_ri4/1
  Gateway Out27/1 -> Scope6/1
  Gateway Out28/1 -> Scope6/2
  Gateway Out26/1 -> Scope6/3
  Gateway Out29/1 -> Scope6/4
  Gateway Out30/1 -> Scope6/5
  Reinterpret8/1 -> Gateway Out28/1
  Reinterpret9/1 -> Gateway Out26/1
  Reinterpret10/1 -> Gateway Out29/1
  Reinterpret11/1 -> Gateway Out30/1
  sim_cns1/1 -> adc/2
  Constant6/1 -> reg_output_bitselect_pol2/1
  reg_output_bitselect_pol2/1 -> Slice10/1
  Slice10/1 -> bitselect_5/1(bitselect), bitselect_6/1(bitselect), bitselect_7/1(bitselect), bitselect_8/1(bitselect)
  pfb_fir_real/2(pol1_out1) -> fft_wideband_real/3(in0)
  pfb_fir_real/3(pol1_out2) -> fft_wideband_real/4(in1)
  pfb_fir_real/4(pol1_out3) -> fft_wideband_real/5(in2)
  pfb_fir_real/5(pol1_out4) -> fft_wideband_real/6(in3)
  pfb_fir_real1/2(pol1_out1) -> fft_wideband_real1/3(in0)
  pfb_fir_real1/3(pol1_out2) -> fft_wideband_real1/4(in1)
  pfb_fir_real1/4(pol1_out3) -> fft_wideband_real1/5(in2)
  pfb_fir_real1/5(pol1_out4) -> fft_wideband_real1/6(in3)
  pfb_fir_real/1(sync_out) -> fft_wideband_real/1(sync)
  pfb_fir_real1/1(sync_out) -> fft_wideband_real1/1(sync)
  fft_delay/1 -> coeff_delay/1
  pipeline/1(q) -> scale1/2(data_in)
  pipeline1/1(q) -> scale2/2(data_in)
  pipeline2/1(q) -> scale3/2(data_in)
  pipeline3/1(q) -> scale4/2(data_in)
  adc_data_delay1/1 -> force_adc_i0/1, pfb_fir_real/2(pol1_in1)
  adc_data_delay2/1 -> force_adc_i1/1, pfb_fir_real/3(pol1_in2)
  adc_data_delay3/1 -> force_adc_i2/1, pfb_fir_real/4(pol1_in3)
  adc_data_delay4/1 -> force_adc_i3/1, pfb_fir_real/5(pol1_in4)
  adc_data_delay5/1 -> force_adc_i8/1, pfb_fir_real1/2(pol1_in1)
  adc_data_delay6/1 -> force_adc_i9/1, pfb_fir_real1/3(pol1_in2)
  adc_data_delay7/1 -> force_adc_i10/1, pfb_fir_real1/4(pol1_in3)
  adc_data_delay8/1 -> force_adc_i11/1, pfb_fir_real1/5(pol1_in4)
  system_counter/1 -> bram_adcscope_2/1
  Concat1/1 -> bram_adcscope_2/2
  adc/9 -> Terminator1/1
  adc/10 -> Terminator3/1
  led2_pps/1 -> Terminator7/1
  adc/11 -> Terminator4/1
  adc/12 -> Terminator6/1
  adc/17 -> Terminator5/1
  cnt_adcscope_1/3 -> Terminator8/1
  bram_adcscope_1/1 -> Terminator11/1
  cnt_adcscope_2/3 -> Terminator9/1
  bram_adcscope_3/1 -> Terminator2/1
  Gateway Out4/1 -> Scope1/1
  Gateway Out5/1 -> Scope1/2
  fft_wideband_real/3(out1) -> pipeline1/1(d)
  fft_wideband_real1/3(out1) -> pipeline3/1(d)
  reorder/1(sync_out) -> square_transposer/1
  square_transposer/1 -> bram_adcscope_2/3
  cns_reorder/1 -> reorder/2(en)
  cram0/1 -> reorder/3(din0)
  cram1/1 -> reorder/4(din1)
  reorder/3(dout0) -> square_transposer/2
  reorder/4(dout1) -> square_transposer/3
  square_transposer/2 -> Concat1/1
  square_transposer/3 -> Concat1/2
  force_adc_i8/1 -> concat_adcscope_3/1
  Constant/1 -> scale1/1(coeff)
  Constant1/1 -> scale2/1(coeff)
  Constant3/1 -> scale3/1(coeff)
  Constant4/1 -> scale4/1(coeff)

== system pasp_16i16c128s_part/bitselect_1 ==
  bitselect : Inport  OutDataType=sfix(16)  OutScaling=2^0
  data_in : Inport  Port=2  OutDataType=sfix(16)  OutScaling=2^0
  Convert_{1..4} (4 alike) : xbsIndex_r4/Convert  arith_type=Unsigned  n_bits=8  bin_pt=0  quantization=Truncate  overflow=Saturate  latency=0  dbl_ovrd=off  pipeline=off  block_type=convert
      settings: xl_area=[0, 0, 0, 0, 0, 0, 0]  block_version=10.1.3
  mux_bitselect : xbsIndex_r4/Mux  inputs=4  en=off  latency=1  precision=Full  arith_type="Signed  (2's comp)"  n_bits=8  bin_pt=2  quantization=Truncate  overflow=Wrap  dbl_ovrd=off  block_type=mux
      settings: xl_area=[0, 0, 0, 0, 0, 0, 0]
  reint_bp_0 : xbsIndex_r4/Reinterpret  force_arith_type=on  arith_type=Unsigned  force_bin_pt=on  bin_pt=0  block_type=reinterpret
      settings: infoedit="Changes signal type without altering the binary representation.   You can changed the signal between signed and unsigned, and relocate the binary point.<P><P>Hardware notes: In hardware this block costs nothing.<P><P>Example:  Suppose the input is 6 bits wide, signed, with 2 fractional bits, and the output is forced to unsigned with 0 fractional bits.  Then an input of -2.0 (1110.00 in binary 2's complement) becomes an output of 56 (111000 in binary)."  block_version=10.1.3
  slc_bitselect_1 : xbsIndex_r4/Slice  nbits=18  boolean_output=off  mode=Lower Bit Location + Width  bit1=0  base1=MSB of Input  bit0=0  base0=LSB of Input  dbl_ovrd=off  block_type=slice
      settings: infoedit="Extracts a given range of bits from each input sample and presents it at the output.  The output type is ordinarily unsigned with binary point at zero, but can be Boolean when the slice is one bit wide.<P><P>Hardware notes: In hardware this block costs nothing."  block_version=10.1.3
  slc_bitselect_2 : xbsIndex_r4/Slice  nbits=14  boolean_output=off  mode=Lower Bit Location + Width  bit1=0  base1=MSB of Input  bit0=4  base0=LSB of Input  dbl_ovrd=off  block_type=slice
      settings: infoedit="Extracts a given range of bits from each input sample and presents it at the output.  The output type is ordinarily unsigned with binary point at zero, but can be Boolean when the slice is one bit wide.<P><P>Hardware notes: In hardware this block costs nothing."  block_version=10.1.3
  slc_bitselect_3 : xbsIndex_r4/Slice  nbits=10  boolean_output=off  mode=Lower Bit Location + Width  bit1=0  base1=MSB of Input  bit0=8  base0=LSB of Input  dbl_ovrd=off  block_type=slice
      settings: infoedit="Extracts a given range of bits from each input sample and presents it at the output.  The output type is ordinarily unsigned with binary point at zero, but can be Boolean when the slice is one bit wide.<P><P>Hardware notes: In hardware this block costs nothing."  block_version=10.1.3
  slc_bitselect_4 : xbsIndex_r4/Slice  nbits=8  boolean_output=off  mode=Lower Bit Location + Width  bit1=0  base1=MSB of Input  bit0=10  base0=LSB of Input  dbl_ovrd=off  block_type=slice
      settings: infoedit="Extracts a given range of bits from each input sample and presents it at the output.  The output type is ordinarily unsigned with binary point at zero, but can be Boolean when the slice is one bit wide.<P><P>Hardware notes: In hardware this block costs nothing."  block_version=10.1.3
  data_out : Outport  OutDataType=sfix(16)  OutScaling=2^0
  wires:
  slc_bitselect_1/1 -> Convert_1/1
  slc_bitselect_2/1 -> Convert_2/1
  slc_bitselect_3/1 -> Convert_3/1
  slc_bitselect_4/1 -> Convert_4/1
  data_in/1 -> reint_bp_0/1
  bitselect/1 -> mux_bitselect/1
  mux_bitselect/1 -> data_out/1
  reint_bp_0/1 -> slc_bitselect_4/1, slc_bitselect_3/1, slc_bitselect_2/1, slc_bitselect_1/1
  Convert_1/1 -> mux_bitselect/2
  Convert_2/1 -> mux_bitselect/3
  Convert_3/1 -> mux_bitselect/4
  Convert_4/1 -> mux_bitselect/5

== system pasp_16i16c128s_part/bitselect_2 ==
  bitselect : Inport  OutDataType=sfix(16)  OutScaling=2^0
  data_in : Inport  Port=2  OutDataType=sfix(16)  OutScaling=2^0
  Convert_{1..4} (4 alike) : xbsIndex_r4/Convert  arith_type=Unsigned  n_bits=8  bin_pt=0  quantization=Truncate  overflow=Saturate  latency=0  dbl_ovrd=off  pipeline=off  block_type=convert
      settings: xl_area=[0, 0, 0, 0, 0, 0, 0]  block_version=10.1.3
  mux_bitselect : xbsIndex_r4/Mux  inputs=4  en=off  latency=1  precision=Full  arith_type="Signed  (2's comp)"  n_bits=8  bin_pt=2  quantization=Truncate  overflow=Wrap  dbl_ovrd=off  block_type=mux
      settings: xl_area=[0, 0, 0, 0, 0, 0, 0]
  reint_bp_0 : xbsIndex_r4/Reinterpret  force_arith_type=on  arith_type=Unsigned  force_bin_pt=on  bin_pt=0  block_type=reinterpret
      settings: infoedit="Changes signal type without altering the binary representation.   You can changed the signal between signed and unsigned, and relocate the binary point.<P><P>Hardware notes: In hardware this block costs nothing.<P><P>Example:  Suppose the input is 6 bits wide, signed, with 2 fractional bits, and the output is forced to unsigned with 0 fractional bits.  Then an input of -2.0 (1110.00 in binary 2's complement) becomes an output of 56 (111000 in binary)."  block_version=10.1.3
  slc_bitselect_1 : xbsIndex_r4/Slice  nbits=18  boolean_output=off  mode=Lower Bit Location + Width  bit1=0  base1=MSB of Input  bit0=0  base0=LSB of Input  dbl_ovrd=off  block_type=slice
      settings: infoedit="Extracts a given range of bits from each input sample and presents it at the output.  The output type is ordinarily unsigned with binary point at zero, but can be Boolean when the slice is one bit wide.<P><P>Hardware notes: In hardware this block costs nothing."  block_version=10.1.3
  slc_bitselect_2 : xbsIndex_r4/Slice  nbits=14  boolean_output=off  mode=Lower Bit Location + Width  bit1=0  base1=MSB of Input  bit0=4  base0=LSB of Input  dbl_ovrd=off  block_type=slice
      settings: infoedit="Extracts a given range of bits from each input sample and presents it at the output.  The output type is ordinarily unsigned with binary point at zero, but can be Boolean when the slice is one bit wide.<P><P>Hardware notes: In hardware this block costs nothing."  block_version=10.1.3
  slc_bitselect_3 : xbsIndex_r4/Slice  nbits=10  boolean_output=off  mode=Lower Bit Location + Width  bit1=0  base1=MSB of Input  bit0=8  base0=LSB of Input  dbl_ovrd=off  block_type=slice
      settings: infoedit="Extracts a given range of bits from each input sample and presents it at the output.  The output type is ordinarily unsigned with binary point at zero, but can be Boolean when the slice is one bit wide.<P><P>Hardware notes: In hardware this block costs nothing."  block_version=10.1.3
  slc_bitselect_4 : xbsIndex_r4/Slice  nbits=8  boolean_output=off  mode=Lower Bit Location + Width  bit1=0  base1=MSB of Input  bit0=10  base0=LSB of Input  dbl_ovrd=off  block_type=slice
      settings: infoedit="Extracts a given range of bits from each input sample and presents it at the output.  The output type is ordinarily unsigned with binary point at zero, but can be Boolean when the slice is one bit wide.<P><P>Hardware notes: In hardware this block costs nothing."  block_version=10.1.3
  data_out : Outport  OutDataType=sfix(16)  OutScaling=2^0
  wires:
  slc_bitselect_1/1 -> Convert_1/1
  slc_bitselect_2/1 -> Convert_2/1
  slc_bitselect_3/1 -> Convert_3/1
  slc_bitselect_4/1 -> Convert_4/1
  data_in/1 -> reint_bp_0/1
  bitselect/1 -> mux_bitselect/1
  mux_bitselect/1 -> data_out/1
  reint_bp_0/1 -> slc_bitselect_4/1, slc_bitselect_3/1, slc_bitselect_2/1, slc_bitselect_1/1
  Convert_1/1 -> mux_bitselect/2
  Convert_2/1 -> mux_bitselect/3
  Convert_3/1 -> mux_bitselect/4
  Convert_4/1 -> mux_bitselect/5
